simulink library "inp"  (Simulink 8.2)
settings: Solver=ode45  SolverMode=SingleTasking  StartTime=0.0  StopTime=10.0  RelTol=1e-3
undefined symbols (not defined in the file or in its folder): C_TICK_TIME_S, ENUM_FAULTSTATE_FAIL, ENUM_FAULTSTATE_INDETERMINATE, ENUM_FAULTSTATE_PASS, ENUM_KEYSWITCH_ON, FALSE, KINP_ABS1CANCommuOk_flg_ovrdflg, KINP_ABS1CANCommuOk_flg_ovrdval, KINP_ABS1CANPeriodTime_s, KINP_ABS1CANRolCntOk_flg_ovrdflg, KINP_ABS1CANRolCntOk_flg_ovrdval, KINP_ABS2CANChkSumOk_flg_ovrdflg, KINP_ABS2CANChkSumOk_flg_ovrdval, KINP_ABS2CANCommuOk_flg_ovrdflg, KINP_ABS2CANCommuOk_flg_ovrdval, KINP_ABS2CANPeriodTime_s, KINP_ABS2CANRolCntOk_flg_ovrdflg, KINP_ABS2CANRolCntOk_flg_ovrdval, KINP_ABS3CANCommuOk_flg_ovrdflg, KINP_ABS3CANCommuOk_flg_ovrdval, KINP_ABS4CANCommuOk_flg_ovrdflg, KINP_ABS4CANCommuOk_flg_ovrdval, KINP_ABSActive_flg_defval, KINP_ABSActive_flg_invalid, KINP_ABSActive_flg_ovrdflg, KINP_ABSActive_flg_ovrdval, KINP_ABSActive_flg_setvalid, KINP_ABSActive_flg_srvcflg, KINP_ABSActive_flg_srvcval, KINP_ABSCANAlive_flg_ovrdflg, KINP_ABSCANAlive_flg_ovrdval, KINP_ABSCANCommuOk_flg_ovrdflg, KINP_ABSCANCommuOk_flg_ovrdval, KINP_ABSCANValidOk_flg_ovrdflg, KINP_ABSCANValidOk_flg_ovrdval, KINP_ABSFault_flg_defval, KINP_ABSFault_flg_invalid, KINP_ABSFault_flg_ovrdflg, KINP_ABSFault_flg_ovrdval, KINP_ABSFault_flg_setvalid, KINP_ABSFault_flg_srvcflg, KINP_ABSFault_flg_srvcval, KINP_AC1CANCommuOk_flg_ovrdflg, KINP_AC1CANCommuOk_flg_ovrdval, KINP_ACCANAlive_flg_ovrdflg, KINP_ACCANAlive_flg_ovrdval, KINP_ACCANCommuOk_flg_ovrdflg, KINP_ACCANCommuOk_flg_ovrdval, KINP_ACCANValidOk_flg_ovrdflg, KINP_ACCANValidOk_flg_ovrdval, KINP_ACCCancelH_flg_defval, KINP_ACCCancelH_flg_invalid, KINP_ACCCancelH_flg_ovrdflg, KINP_ACCCancelH_flg_ovrdval, KINP_ACCCancelH_flg_setvalid, KINP_ACCCancelH_flg_srvcflg, KINP_ACCCancelH_flg_srvcval, KINP_AccelPedalPosn_pct_defval, KINP_AccelPedalPosn_pct_gain, KINP_AccelPedalPosn_pct_invalid ... (+1866)
libraries: inp x192, hcu_lib x34, simulink x33
(part 20 of 21)

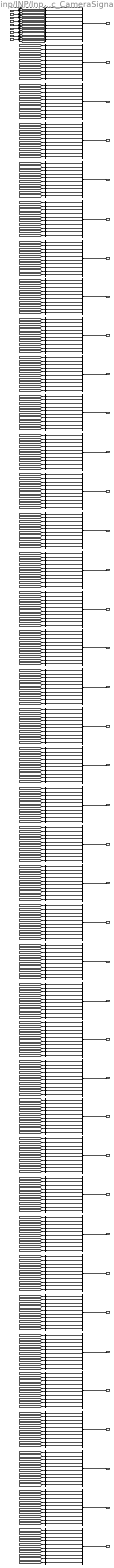
== system inp/INP/InputSignals/SignalProcess/Proc_CameraSignals ==
  VINP_Obstacle1_BUS_InHAL : Inport
  VINP_Obstacle2_BUS_InHAL : Inport  Port=2
  VINP_Obstacle3_BUS_InHAL : Inport  Port=3
  VINP_Obstacle4_BUS_InHAL : Inport  Port=4
  VINP_Obstacle5_BUS_InHAL : Inport  Port=5
  VINP_Obstacle6_BUS_InHAL : Inport  Port=6
  VINP_Obstacle7_BUS_InHAL : Inport  Port=7
  VINP_Obstacle8_BUS_InHAL : Inport  Port=8
  VINP_Obstacle9_BUS_InHAL : Inport  Port=9
  VINP_Obstacle10_BUS_InHAL : Inport  Port=10
  Bus Selector : BusSelector  OutputSignals=VINP_CamObs1ID_num_InHAL
  Bus Selector1 : BusSelector  OutputSignals=VINP_CamObs2ID_num_InHAL
  Bus Selector10 : BusSelector  OutputSignals=VINP_CamObs1IDValid_flg_InHAL
  Bus Selector100 : BusSelector  OutputSignals=VINP_CamObs1Status_enum_InHAL
  Bus Selector101 : BusSelector  OutputSignals=VINP_CamObs2Status_enum_InHAL
  Bus Selector102 : BusSelector  OutputSignals=VINP_CamObs3Status_enum_InHAL
  Bus Selector103 : BusSelector  OutputSignals=VINP_CamObs4Status_enum_InHAL
  Bus Selector104 : BusSelector  OutputSignals=VINP_CamObs5Status_enum_InHAL
  Bus Selector105 : BusSelector  OutputSignals=VINP_CamObs6Status_enum_InHAL
  Bus Selector106 : BusSelector  OutputSignals=VINP_CamObs7Status_enum_InHAL
  Bus Selector107 : BusSelector  OutputSignals=VINP_CamObs8Status_enum_InHAL
  Bus Selector108 : BusSelector  OutputSignals=VINP_CamObs9Status_enum_InHAL
  Bus Selector109 : BusSelector  OutputSignals=VINP_CamObs10Status_enum_InHAL
  Bus Selector11 : BusSelector  OutputSignals=VINP_CamObs2IDValid_flg_InHAL
  Bus Selector110 : BusSelector  OutputSignals=VINP_CamObs1StatusValid_flg_InHAL
  Bus Selector111 : BusSelector  OutputSignals=VINP_CamObs2StatusValid_flg_InHAL
  Bus Selector112 : BusSelector  OutputSignals=VINP_CamObs3StatusValid_flg_InHAL
  Bus Selector113 : BusSelector  OutputSignals=VINP_CamObs4StatusValid_flg_InHAL
  Bus Selector114 : BusSelector  OutputSignals=VINP_CamObs5StatusValid_flg_InHAL
  Bus Selector115 : BusSelector  OutputSignals=VINP_CamObs6StatusValid_flg_InHAL
  Bus Selector116 : BusSelector  OutputSignals=VINP_CamObs7StatusValid_flg_InHAL
  Bus Selector117 : BusSelector  OutputSignals=VINP_CamObs8StatusValid_flg_InHAL
  Bus Selector118 : BusSelector  OutputSignals=VINP_CamObs9StatusValid_flg_InHAL
  Bus Selector119 : BusSelector  OutputSignals=VINP_CamObs10StatusValid_flg_InHAL
  Bus Selector12 : BusSelector  OutputSignals=VINP_CamObs3IDValid_flg_InHAL
  Bus Selector120 : BusSelector  OutputSignals=VINP_CamObs1Valid_enum_InHAL
  Bus Selector121 : BusSelector  OutputSignals=VINP_CamObs2Valid_enum_InHAL
  Bus Selector122 : BusSelector  OutputSignals=VINP_CamObs3Valid_enum_InHAL
  Bus Selector123 : BusSelector  OutputSignals=VINP_CamObs4Valid_enum_InHAL
  Bus Selector124 : BusSelector  OutputSignals=VINP_CamObs5Valid_enum_InHAL
  Bus Selector125 : BusSelector  OutputSignals=VINP_CamObs6Valid_enum_InHAL
  Bus Selector126 : BusSelector  OutputSignals=VINP_CamObs7Valid_enum_InHAL
  Bus Selector127 : BusSelector  OutputSignals=VINP_CamObs8Valid_enum_InHAL
  Bus Selector128 : BusSelector  OutputSignals=VINP_CamObs9Valid_enum_InHAL
  Bus Selector129 : BusSelector  OutputSignals=VINP_CamObs10Valid_enum_InHAL
  Bus Selector13 : BusSelector  OutputSignals=VINP_CamObs4IDValid_flg_InHAL
  Bus Selector130 : BusSelector  OutputSignals=VINP_CamObs1ValidValid_flg_InHAL
  Bus Selector131 : BusSelector  OutputSignals=VINP_CamObs2ValidValid_flg_InHAL
  Bus Selector132 : BusSelector  OutputSignals=VINP_CamObs3ValidValid_flg_InHAL
  Bus Selector133 : BusSelector  OutputSignals=VINP_CamObs4ValidValid_flg_InHAL
  Bus Selector134 : BusSelector  OutputSignals=VINP_CamObs5ValidValid_flg_InHAL
  Bus Selector135 : BusSelector  OutputSignals=VINP_CamObs6ValidValid_flg_InHAL
  Bus Selector136 : BusSelector  OutputSignals=VINP_CamObs7ValidValid_flg_InHAL
  Bus Selector137 : BusSelector  OutputSignals=VINP_CamObs8ValidValid_flg_InHAL
  Bus Selector138 : BusSelector  OutputSignals=VINP_CamObs9ValidValid_flg_InHAL
  Bus Selector139 : BusSelector  OutputSignals=VINP_CamObs10ValidValid_flg_InHAL
  Bus Selector14 : BusSelector  OutputSignals=VINP_CamObs5IDValid_flg_InHAL
  Bus Selector140 : BusSelector  OutputSignals=VINP_CamObs1MoveInO_enum_InHAL
  Bus Selector141 : BusSelector  OutputSignals=VINP_CamObs2MoveInO_enum_InHAL
  Bus Selector142 : BusSelector  OutputSignals=VINP_CamObs3MoveInO_enum_InHAL
  Bus Selector143 : BusSelector  OutputSignals=VINP_CamObs4MoveInO_enum_InHAL
  Bus Selector144 : BusSelector  OutputSignals=VINP_CamObs5MoveInO_enum_InHAL
  Bus Selector145 : BusSelector  OutputSignals=VINP_CamObs6MoveInO_enum_InHAL
  Bus Selector146 : BusSelector  OutputSignals=VINP_CamObs7MoveInO_enum_InHAL
  Bus Selector147 : BusSelector  OutputSignals=VINP_CamObs8MoveInO_enum_InHAL
  Bus Selector148 : BusSelector  OutputSignals=VINP_CamObs9MoveInO_enum_InHAL
  Bus Selector149 : BusSelector  OutputSignals=VINP_CamObs10MoveInO_enum_InHAL
  Bus Selector15 : BusSelector  OutputSignals=VINP_CamObs6IDValid_flg_InHAL
  Bus Selector150 : BusSelector  OutputSignals=VINP_CamObs1MoveInOValid_flg_InHAL
  Bus Selector151 : BusSelector  OutputSignals=VINP_CamObs2MoveInOValid_flg_InHAL
  Bus Selector152 : BusSelector  OutputSignals=VINP_CamObs3MoveInOValid_flg_InHAL
  Bus Selector153 : BusSelector  OutputSignals=VINP_CamObs4MoveInOValid_flg_InHAL
  Bus Selector154 : BusSelector  OutputSignals=VINP_CamObs5MoveInOValid_flg_InHAL
  Bus Selector155 : BusSelector  OutputSignals=VINP_CamObs6MoveInOValid_flg_InHAL
  Bus Selector156 : BusSelector  OutputSignals=VINP_CamObs7MoveInOValid_flg_InHAL
  Bus Selector157 : BusSelector  OutputSignals=VINP_CamObs8MoveInOValid_flg_InHAL
  Bus Selector158 : BusSelector  OutputSignals=VINP_CamObs9MoveInOValid_flg_InHAL
  Bus Selector159 : BusSelector  OutputSignals=VINP_CamObs10MoveInOValid_flg_InHAL
  Bus Selector16 : BusSelector  OutputSignals=VINP_CamObs7IDValid_flg_InHAL
  Bus Selector160 : BusSelector  OutputSignals=VINP_CamObs1Blinker_enum_InHAL
  Bus Selector161 : BusSelector  OutputSignals=VINP_CamObs2Blinker_enum_InHAL
  Bus Selector162 : BusSelector  OutputSignals=VINP_CamObs3Blinker_enum_InHAL
  Bus Selector163 : BusSelector  OutputSignals=VINP_CamObs4Blinker_enum_InHAL
  Bus Selector164 : BusSelector  OutputSignals=VINP_CamObs5Blinker_enum_InHAL
  Bus Selector165 : BusSelector  OutputSignals=VINP_CamObs6Blinker_enum_InHAL
  Bus Selector166 : BusSelector  OutputSignals=VINP_CamObs7Blinker_enum_InHAL
  Bus Selector167 : BusSelector  OutputSignals=VINP_CamObs8Blinker_enum_InHAL
  Bus Selector168 : BusSelector  OutputSignals=VINP_CamObs9Blinker_enum_InHAL
  Bus Selector169 : BusSelector  OutputSignals=VINP_CamObs10Blinker_enum_InHAL
  Bus Selector17 : BusSelector  OutputSignals=VINP_CamObs8IDValid_flg_InHAL
  Bus Selector170 : BusSelector  OutputSignals=VINP_CamObs1BlinkerValid_flg_InHAL
  Bus Selector171 : BusSelector  OutputSignals=VINP_CamObs2BlinkerValid_flg_InHAL
  Bus Selector172 : BusSelector  OutputSignals=VINP_CamObs3BlinkerValid_flg_InHAL
  Bus Selector173 : BusSelector  OutputSignals=VINP_CamObs4BlinkerValid_flg_InHAL
  Bus Selector174 : BusSelector  OutputSignals=VINP_CamObs5BlinkerValid_flg_InHAL
  Bus Selector175 : BusSelector  OutputSignals=VINP_CamObs6BlinkerValid_flg_InHAL
  Bus Selector176 : BusSelector  OutputSignals=VINP_CamObs7BlinkerValid_flg_InHAL
  Bus Selector177 : BusSelector  OutputSignals=VINP_CamObs8BlinkerValid_flg_InHAL
  Bus Selector178 : BusSelector  OutputSignals=VINP_CamObs9BlinkerValid_flg_InHAL
  Bus Selector179 : BusSelector  OutputSignals=VINP_CamObs10BlinkerValid_flg_InHAL
  Bus Selector18 : BusSelector  OutputSignals=VINP_CamObs9IDValid_flg_InHAL
  Bus Selector180 : BusSelector  OutputSignals=VINP_CamObs1BrakeLight_flg_InHAL
  Bus Selector181 : BusSelector  OutputSignals=VINP_CamObs2BrakeLight_flg_InHAL
  Bus Selector182 : BusSelector  OutputSignals=VINP_CamObs3BrakeLight_flg_InHAL
  Bus Selector183 : BusSelector  OutputSignals=VINP_CamObs4BrakeLight_flg_InHAL
  Bus Selector184 : BusSelector  OutputSignals=VINP_CamObs5BrakeLight_flg_InHAL
  Bus Selector185 : BusSelector  OutputSignals=VINP_CamObs6BrakeLight_flg_InHAL
  Bus Selector186 : BusSelector  OutputSignals=VINP_CamObs7BrakeLight_flg_InHAL
  Bus Selector187 : BusSelector  OutputSignals=VINP_CamObs8BrakeLight_flg_InHAL
  Bus Selector188 : BusSelector  OutputSignals=VINP_CamObs9BrakeLight_flg_InHAL
  Bus Selector189 : BusSelector  OutputSignals=VINP_CamObs10BrakeLight_flg_InHAL
  Bus Selector19 : BusSelector  OutputSignals=VINP_CamObs10IDValid_flg_InHAL
  Bus Selector190 : BusSelector  OutputSignals=VINP_CamObs1BrakeLightValid_flg_InHAL
  Bus Selector191 : BusSelector  OutputSignals=VINP_CamObs2BrakeLightValid_flg_InHAL
  Bus Selector192 : BusSelector  OutputSignals=VINP_CamObs3BrakeLightValid_flg_InHAL
  Bus Selector193 : BusSelector  OutputSignals=VINP_CamObs4BrakeLightValid_flg_InHAL
  Bus Selector194 : BusSelector  OutputSignals=VINP_CamObs5BrakeLightValid_flg_InHAL
  Bus Selector195 : BusSelector  OutputSignals=VINP_CamObs6BrakeLightValid_flg_InHAL
  Bus Selector196 : BusSelector  OutputSignals=VINP_CamObs7BrakeLightValid_flg_InHAL
  Bus Selector197 : BusSelector  OutputSignals=VINP_CamObs8BrakeLightValid_flg_InHAL
  Bus Selector198 : BusSelector  OutputSignals=VINP_CamObs9BrakeLightValid_flg_InHAL
  Bus Selector199 : BusSelector  OutputSignals=VINP_CamObs10BrakeLightValid_flg_InHAL
  Bus Selector2 : BusSelector  OutputSignals=VINP_CamObs3ID_num_InHAL
  Bus Selector20 : BusSelector  OutputSignals=VINP_CamObs1PosX_m_InHAL
  Bus Selector200 : BusSelector  OutputSignals=VINP_CamObs1Length_m_InHAL
  Bus Selector201 : BusSelector  OutputSignals=VINP_CamObs1CIPVValid_flg_InHAL
  Bus Selector202 : BusSelector  OutputSignals=VINP_CamObs2CIPVValid_flg_InHAL
  Bus Selector203 : BusSelector  OutputSignals=VINP_CamObs3CIPVValid_flg_InHAL
  Bus Selector204 : BusSelector  OutputSignals=VINP_CamObs4CIPVValid_flg_InHAL
  Bus Selector205 : BusSelector  OutputSignals=VINP_CamObs5CIPVValid_flg_InHAL
  Bus Selector206 : BusSelector  OutputSignals=VINP_CamObs6CIPVValid_flg_InHAL
  Bus Selector207 : BusSelector  OutputSignals=VINP_CamObs7CIPVValid_flg_InHAL
  Bus Selector208 : BusSelector  OutputSignals=VINP_CamObs8CIPVValid_flg_InHAL
  Bus Selector209 : BusSelector  OutputSignals=VINP_CamObs9CIPVValid_flg_InHAL
  Bus Selector21 : BusSelector  OutputSignals=VINP_CamObs2PosX_m_InHAL
  Bus Selector210 : BusSelector  OutputSignals=VINP_CamObs10CIPVValid_flg_InHAL
  Bus Selector211 : BusSelector  OutputSignals=VINP_CamObs2Length_m_InHAL
  Bus Selector212 : BusSelector  OutputSignals=VINP_CamObs1VelY_mps_InHAL
  Bus Selector213 : BusSelector  OutputSignals=VINP_CamObs2VelY_mps_InHAL
  Bus Selector214 : BusSelector  OutputSignals=VINP_CamObs3VelY_mps_InHAL
  Bus Selector215 : BusSelector  OutputSignals=VINP_CamObs4VelY_mps_InHAL
  Bus Selector216 : BusSelector  OutputSignals=VINP_CamObs5VelY_mps_InHAL
  Bus Selector217 : BusSelector  OutputSignals=VINP_CamObs6VelY_mps_InHAL
  Bus Selector218 : BusSelector  OutputSignals=VINP_CamObs7VelY_mps_InHAL
  Bus Selector219 : BusSelector  OutputSignals=VINP_CamObs8VelY_mps_InHAL
  Bus Selector22 : BusSelector  OutputSignals=VINP_CamObs3PosX_m_InHAL
  Bus Selector220 : BusSelector  OutputSignals=VINP_CamObs9VelY_mps_InHAL
  Bus Selector221 : BusSelector  OutputSignals=VINP_CamObs10VelY_mps_InHAL
  Bus Selector222 : BusSelector  OutputSignals=VINP_CamObs3Length_m_InHAL
  Bus Selector223 : BusSelector  OutputSignals=VINP_CamObs1VelYValid_flg_InHAL
  Bus Selector224 : BusSelector  OutputSignals=VINP_CamObs2VelYValid_flg_InHAL
  Bus Selector225 : BusSelector  OutputSignals=VINP_CamObs3VelYValid_flg_InHAL
  Bus Selector226 : BusSelector  OutputSignals=VINP_CamObs4VelYValid_flg_InHAL
  Bus Selector227 : BusSelector  OutputSignals=VINP_CamObs5VelYValid_flg_InHAL
  Bus Selector228 : BusSelector  OutputSignals=VINP_CamObs6VelYValid_flg_InHAL
  Bus Selector229 : BusSelector  OutputSignals=VINP_CamObs7VelYValid_flg_InHAL
  Bus Selector23 : BusSelector  OutputSignals=VINP_CamObs4PosX_m_InHAL
  Bus Selector230 : BusSelector  OutputSignals=VINP_CamObs8VelYValid_flg_InHAL
  Bus Selector231 : BusSelector  OutputSignals=VINP_CamObs9VelYValid_flg_InHAL
  Bus Selector232 : BusSelector  OutputSignals=VINP_CamObs10VelYValid_flg_InHAL
  Bus Selector233 : BusSelector  OutputSignals=VINP_CamObs4Length_m_InHAL
  Bus Selector234 : BusSelector  OutputSignals=VINP_CamObs1AccX_mps2_InHAL
  Bus Selector235 : BusSelector  OutputSignals=VINP_CamObs2AccX_mps2_InHAL
  Bus Selector236 : BusSelector  OutputSignals=VINP_CamObs3AccX_mps2_InHAL
  Bus Selector237 : BusSelector  OutputSignals=VINP_CamObs4AccX_mps2_InHAL
  Bus Selector238 : BusSelector  OutputSignals=VINP_CamObs5AccX_mps2_InHAL
  Bus Selector239 : BusSelector  OutputSignals=VINP_CamObs6AccX_mps2_InHAL
  Bus Selector24 : BusSelector  OutputSignals=VINP_CamObs5PosX_m_InHAL
  Bus Selector240 : BusSelector  OutputSignals=VINP_CamObs7AccX_mps2_InHAL
  Bus Selector241 : BusSelector  OutputSignals=VINP_CamObs8AccX_mps2_InHAL
  Bus Selector242 : BusSelector  OutputSignals=VINP_CamObs9AccX_mps2_InHAL
  Bus Selector243 : BusSelector  OutputSignals=VINP_CamObs10AccX_mps2_InHAL
  Bus Selector244 : BusSelector  OutputSignals=VINP_CamObs5Length_m_InHAL
  Bus Selector245 : BusSelector  OutputSignals=VINP_CamObs1AccXValid_flg_InHAL
  Bus Selector246 : BusSelector  OutputSignals=VINP_CamObs2AccXValid_flg_InHAL
  Bus Selector247 : BusSelector  OutputSignals=VINP_CamObs3AccXValid_flg_InHAL
  Bus Selector248 : BusSelector  OutputSignals=VINP_CamObs4AccXValid_flg_InHAL
  Bus Selector249 : BusSelector  OutputSignals=VINP_CamObs5AccXValid_flg_InHAL
  Bus Selector25 : BusSelector  OutputSignals=VINP_CamObs6PosX_m_InHAL
  Bus Selector250 : BusSelector  OutputSignals=VINP_CamObs6AccXValid_flg_InHAL
  Bus Selector251 : BusSelector  OutputSignals=VINP_CamObs7AccXValid_flg_InHAL
  Bus Selector252 : BusSelector  OutputSignals=VINP_CamObs8AccXValid_flg_InHAL
  Bus Selector253 : BusSelector  OutputSignals=VINP_CamObs9AccXValid_flg_InHAL
  Bus Selector254 : BusSelector  OutputSignals=VINP_CamObs10AccXValid_flg_InHAL
  Bus Selector255 : BusSelector  OutputSignals=VINP_CamObs6Length_m_InHAL
  Bus Selector256 : BusSelector  OutputSignals=VINP_CamObs1Ang_deg_InHAL
  Bus Selector257 : BusSelector  OutputSignals=VINP_CamObs2Ang_deg_InHAL
  Bus Selector258 : BusSelector  OutputSignals=VINP_CamObs3Ang_deg_InHAL
  Bus Selector259 : BusSelector  OutputSignals=VINP_CamObs4Ang_deg_InHAL
  Bus Selector26 : BusSelector  OutputSignals=VINP_CamObs7PosX_m_InHAL
  Bus Selector260 : BusSelector  OutputSignals=VINP_CamObs5Ang_deg_InHAL
  Bus Selector261 : BusSelector  OutputSignals=VINP_CamObs6Ang_deg_InHAL
  Bus Selector262 : BusSelector  OutputSignals=VINP_CamObs7Ang_deg_InHAL
  Bus Selector263 : BusSelector  OutputSignals=VINP_CamObs8Ang_deg_InHAL
  Bus Selector264 : BusSelector  OutputSignals=VINP_CamObs9Ang_deg_InHAL
  Bus Selector265 : BusSelector  OutputSignals=VINP_CamObs10Ang_deg_InHAL
  Bus Selector266 : BusSelector  OutputSignals=VINP_CamObs7Length_m_InHAL
  Bus Selector267 : BusSelector  OutputSignals=VINP_CamObs1AngValid_flg_InHAL
  Bus Selector268 : BusSelector  OutputSignals=VINP_CamObs2AngValid_flg_InHAL
  Bus Selector269 : BusSelector  OutputSignals=VINP_CamObs3AngValid_flg_InHAL
  Bus Selector27 : BusSelector  OutputSignals=VINP_CamObs8PosX_m_InHAL
  Bus Selector270 : BusSelector  OutputSignals=VINP_CamObs4AngValid_flg_InHAL
  Bus Selector271 : BusSelector  OutputSignals=VINP_CamObs5AngValid_flg_InHAL
  Bus Selector272 : BusSelector  OutputSignals=VINP_CamObs6AngValid_flg_InHAL
  Bus Selector273 : BusSelector  OutputSignals=VINP_CamObs7AngValid_flg_InHAL
  Bus Selector274 : BusSelector  OutputSignals=VINP_CamObs8AngValid_flg_InHAL
  Bus Selector275 : BusSelector  OutputSignals=VINP_CamObs9AngValid_flg_InHAL
  Bus Selector276 : BusSelector  OutputSignals=VINP_CamObs10AngValid_flg_InHAL
  Bus Selector277 : BusSelector  OutputSignals=VINP_CamObs8Length_m_InHAL
  Bus Selector278 : BusSelector  OutputSignals=VINP_CamObs1AngRate_degps_InHAL
  Bus Selector279 : BusSelector  OutputSignals=VINP_CamObs2AngRate_degps_InHAL
  Bus Selector28 : BusSelector  OutputSignals=VINP_CamObs9PosX_m_InHAL
  Bus Selector280 : BusSelector  OutputSignals=VINP_CamObs3AngRate_degps_InHAL
  Bus Selector281 : BusSelector  OutputSignals=VINP_CamObs4AngRate_degps_InHAL
  Bus Selector282 : BusSelector  OutputSignals=VINP_CamObs5AngRate_degps_InHAL
  Bus Selector283 : BusSelector  OutputSignals=VINP_CamObs6AngRate_degps_InHAL
  Bus Selector284 : BusSelector  OutputSignals=VINP_CamObs7AngRate_degps_InHAL
  Bus Selector285 : BusSelector  OutputSignals=VINP_CamObs8AngRate_degps_InHAL
  Bus Selector286 : BusSelector  OutputSignals=VINP_CamObs9AngRate_degps_InHAL
  Bus Selector287 : BusSelector  OutputSignals=VINP_CamObs10AngRate_degps_InHAL
  Bus Selector288 : BusSelector  OutputSignals=VINP_CamObs9Length_m_InHAL
  Bus Selector289 : BusSelector  OutputSignals=VINP_CamObs1AngRateValid_flg_InHAL
  Bus Selector29 : BusSelector  OutputSignals=VINP_CamObs10PosX_m_InHAL
  Bus Selector290 : BusSelector  OutputSignals=VINP_CamObs2AngRateValid_flg_InHAL
  Bus Selector291 : BusSelector  OutputSignals=VINP_CamObs3AngRateValid_flg_InHAL
  Bus Selector292 : BusSelector  OutputSignals=VINP_CamObs4AngRateValid_flg_InHAL
  Bus Selector293 : BusSelector  OutputSignals=VINP_CamObs5AngRateValid_flg_InHAL
  Bus Selector294 : BusSelector  OutputSignals=VINP_CamObs6AngRateValid_flg_InHAL
  Bus Selector295 : BusSelector  OutputSignals=VINP_CamObs7AngRateValid_flg_InHAL
  Bus Selector296 : BusSelector  OutputSignals=VINP_CamObs8AngRateValid_flg_InHAL
  Bus Selector297 : BusSelector  OutputSignals=VINP_CamObs9AngRateValid_flg_InHAL
  Bus Selector298 : BusSelector  OutputSignals=VINP_CamObs10AngRateValid_flg_InHAL
  Bus Selector299 : BusSelector  OutputSignals=VINP_CamObs10Length_m_InHAL
  Bus Selector3 : BusSelector  OutputSignals=VINP_CamObs4ID_num_InHAL
  Bus Selector30 : BusSelector  OutputSignals=VINP_CamObs1PosXValid_flg_InHAL
  Bus Selector300 : BusSelector  OutputSignals=VINP_CamObs1Replaced_flg_InHAL
  Bus Selector301 : BusSelector  OutputSignals=VINP_CamObs2Replaced_flg_InHAL
  Bus Selector302 : BusSelector  OutputSignals=VINP_CamObs3Replaced_flg_InHAL
  Bus Selector303 : BusSelector  OutputSignals=VINP_CamObs4Replaced_flg_InHAL
  Bus Selector304 : BusSelector  OutputSignals=VINP_CamObs5Replaced_flg_InHAL
  Bus Selector305 : BusSelector  OutputSignals=VINP_CamObs6Replaced_flg_InHAL
  Bus Selector306 : BusSelector  OutputSignals=VINP_CamObs7Replaced_flg_InHAL
  Bus Selector307 : BusSelector  OutputSignals=VINP_CamObs8Replaced_flg_InHAL
  Bus Selector308 : BusSelector  OutputSignals=VINP_CamObs9Replaced_flg_InHAL
  Bus Selector309 : BusSelector  OutputSignals=VINP_CamObs10Replaced_flg_InHAL
  Bus Selector31 : BusSelector  OutputSignals=VINP_CamObs2PosXValid_flg_InHAL
  Bus Selector310 : BusSelector  OutputSignals=VINP_CamObs1LengthValid_flg_InHAL
  Bus Selector311 : BusSelector  OutputSignals=VINP_CamObs2LengthValid_flg_InHAL
  Bus Selector312 : BusSelector  OutputSignals=VINP_CamObs3LengthValid_flg_InHAL
  Bus Selector313 : BusSelector  OutputSignals=VINP_CamObs4LengthValid_flg_InHAL
  Bus Selector314 : BusSelector  OutputSignals=VINP_CamObs5LengthValid_flg_InHAL
  Bus Selector315 : BusSelector  OutputSignals=VINP_CamObs6LengthValid_flg_InHAL
  Bus Selector316 : BusSelector  OutputSignals=VINP_CamObs7LengthValid_flg_InHAL
  Bus Selector317 : BusSelector  OutputSignals=VINP_CamObs8LengthValid_flg_InHAL
  Bus Selector318 : BusSelector  OutputSignals=VINP_CamObs9LengthValid_flg_InHAL
  Bus Selector319 : BusSelector  OutputSignals=VINP_CamObs10LengthValid_flg_InHAL
  Bus Selector32 : BusSelector  OutputSignals=VINP_CamObs3PosXValid_flg_InHAL
  Bus Selector320 : BusSelector  OutputSignals=VINP_CamObs1Width_m_InHAL
  Bus Selector321 : BusSelector  OutputSignals=VINP_CamObs2Width_m_InHAL
  Bus Selector322 : BusSelector  OutputSignals=VINP_CamObs3Width_m_InHAL
  Bus Selector323 : BusSelector  OutputSignals=VINP_CamObs4Width_m_InHAL
  Bus Selector324 : BusSelector  OutputSignals=VINP_CamObs5Width_m_InHAL
  Bus Selector325 : BusSelector  OutputSignals=VINP_CamObs6Width_m_InHAL
  Bus Selector326 : BusSelector  OutputSignals=VINP_CamObs7Width_m_InHAL
  Bus Selector327 : BusSelector  OutputSignals=VINP_CamObs8Width_m_InHAL
  Bus Selector328 : BusSelector  OutputSignals=VINP_CamObs9Width_m_InHAL
  Bus Selector329 : BusSelector  OutputSignals=VINP_CamObs10Width_m_InHAL
  Bus Selector33 : BusSelector  OutputSignals=VINP_CamObs4PosXValid_flg_InHAL
  Bus Selector330 : BusSelector  OutputSignals=VINP_CamObs1WidthValid_flg_InHAL
  Bus Selector331 : BusSelector  OutputSignals=VINP_CamObs2WidthValid_flg_InHAL
  Bus Selector332 : BusSelector  OutputSignals=VINP_CamObs3WidthValid_flg_InHAL
  Bus Selector333 : BusSelector  OutputSignals=VINP_CamObs4WidthValid_flg_InHAL
  Bus Selector334 : BusSelector  OutputSignals=VINP_CamObs5WidthValid_flg_InHAL
  Bus Selector335 : BusSelector  OutputSignals=VINP_CamObs6WidthValid_flg_InHAL
  Bus Selector336 : BusSelector  OutputSignals=VINP_CamObs7WidthValid_flg_InHAL
  Bus Selector337 : BusSelector  OutputSignals=VINP_CamObs8WidthValid_flg_InHAL
  Bus Selector338 : BusSelector  OutputSignals=VINP_CamObs9WidthValid_flg_InHAL
  Bus Selector339 : BusSelector  OutputSignals=VINP_CamObs10WidthValid_flg_InHAL
  Bus Selector34 : BusSelector  OutputSignals=VINP_CamObs5PosXValid_flg_InHAL
  Bus Selector340 : BusSelector  OutputSignals=VINP_CamObs1Age_cnt_InHAL
  Bus Selector341 : BusSelector  OutputSignals=VINP_CamObs2Age_cnt_InHAL
  Bus Selector342 : BusSelector  OutputSignals=VINP_CamObs3Age_cnt_InHAL
  Bus Selector343 : BusSelector  OutputSignals=VINP_CamObs4Age_cnt_InHAL
  Bus Selector344 : BusSelector  OutputSignals=VINP_CamObs5Age_cnt_InHAL
  Bus Selector345 : BusSelector  OutputSignals=VINP_CamObs6Age_cnt_InHAL
  Bus Selector346 : BusSelector  OutputSignals=VINP_CamObs7Age_cnt_InHAL
  Bus Selector347 : BusSelector  OutputSignals=VINP_CamObs8Age_cnt_InHAL
  Bus Selector348 : BusSelector  OutputSignals=VINP_CamObs9Age_cnt_InHAL
  Bus Selector349 : BusSelector  OutputSignals=VINP_CamObs10Age_cnt_InHAL
  Bus Selector35 : BusSelector  OutputSignals=VINP_CamObs6PosXValid_flg_InHAL
  Bus Selector350 : BusSelector  OutputSignals=VINP_CamObs1AgeValid_flg_InHAL
  Bus Selector351 : BusSelector  OutputSignals=VINP_CamObs2AgeValid_flg_InHAL
  Bus Selector352 : BusSelector  OutputSignals=VINP_CamObs3AgeValid_flg_InHAL
  Bus Selector353 : BusSelector  OutputSignals=VINP_CamObs4AgeValid_flg_InHAL
  Bus Selector354 : BusSelector  OutputSignals=VINP_CamObs5AgeValid_flg_InHAL
  Bus Selector355 : BusSelector  OutputSignals=VINP_CamObs6AgeValid_flg_InHAL
  Bus Selector356 : BusSelector  OutputSignals=VINP_CamObs7AgeValid_flg_InHAL
  Bus Selector357 : BusSelector  OutputSignals=VINP_CamObs8AgeValid_flg_InHAL
  Bus Selector358 : BusSelector  OutputSignals=VINP_CamObs9AgeValid_flg_InHAL
  Bus Selector359 : BusSelector  OutputSignals=VINP_CamObs10AgeValid_flg_InHAL
  Bus Selector36 : BusSelector  OutputSignals=VINP_CamObs7PosXValid_flg_InHAL
  Bus Selector360 : BusSelector  OutputSignals=VINP_CamObs1Lane_enum_InHAL
  Bus Selector361 : BusSelector  OutputSignals=VINP_CamObs2Lane_enum_InHAL
  Bus Selector362 : BusSelector  OutputSignals=VINP_CamObs3Lane_enum_InHAL
  Bus Selector363 : BusSelector  OutputSignals=VINP_CamObs4Lane_enum_InHAL
  Bus Selector364 : BusSelector  OutputSignals=VINP_CamObs5Lane_enum_InHAL
  Bus Selector365 : BusSelector  OutputSignals=VINP_CamObs6Lane_enum_InHAL
  Bus Selector366 : BusSelector  OutputSignals=VINP_CamObs7Lane_enum_InHAL
  Bus Selector367 : BusSelector  OutputSignals=VINP_CamObs8Lane_enum_InHAL
  Bus Selector368 : BusSelector  OutputSignals=VINP_CamObs9Lane_enum_InHAL
  Bus Selector369 : BusSelector  OutputSignals=VINP_CamObs10Lane_enum_InHAL
  Bus Selector37 : BusSelector  OutputSignals=VINP_CamObs8PosXValid_flg_InHAL
  Bus Selector370 : BusSelector  OutputSignals=VINP_CamObs1LaneValid_flg_InHAL
  Bus Selector371 : BusSelector  OutputSignals=VINP_CamObs2LaneValid_flg_InHAL
  Bus Selector372 : BusSelector  OutputSignals=VINP_CamObs3LaneValid_flg_InHAL
  Bus Selector373 : BusSelector  OutputSignals=VINP_CamObs4LaneValid_flg_InHAL
  Bus Selector374 : BusSelector  OutputSignals=VINP_CamObs5LaneValid_flg_InHAL
  Bus Selector375 : BusSelector  OutputSignals=VINP_CamObs6LaneValid_flg_InHAL
  Bus Selector376 : BusSelector  OutputSignals=VINP_CamObs7LaneValid_flg_InHAL
  Bus Selector377 : BusSelector  OutputSignals=VINP_CamObs8LaneValid_flg_InHAL
  Bus Selector378 : BusSelector  OutputSignals=VINP_CamObs9LaneValid_flg_InHAL
  Bus Selector379 : BusSelector  OutputSignals=VINP_CamObs10LaneValid_flg_InHAL
  Bus Selector38 : BusSelector  OutputSignals=VINP_CamObs9PosXValid_flg_InHAL
  Bus Selector380 : BusSelector  OutputSignals=VINP_CamObs1CIPV_flg_InHAL
  Bus Selector381 : BusSelector  OutputSignals=VINP_CamObs2CIPV_flg_InHAL
  Bus Selector382 : BusSelector  OutputSignals=VINP_CamObs3CIPV_flg_InHAL
  Bus Selector383 : BusSelector  OutputSignals=VINP_CamObs4CIPV_flg_InHAL
  Bus Selector384 : BusSelector  OutputSignals=VINP_CamObs5CIPV_flg_InHAL
  Bus Selector385 : BusSelector  OutputSignals=VINP_CamObs6CIPV_flg_InHAL
  Bus Selector386 : BusSelector  OutputSignals=VINP_CamObs7CIPV_flg_InHAL
  Bus Selector387 : BusSelector  OutputSignals=VINP_CamObs8CIPV_flg_InHAL
  Bus Selector388 : BusSelector  OutputSignals=VINP_CamObs9CIPV_flg_InHAL
  Bus Selector389 : BusSelector  OutputSignals=VINP_CamObs10CIPV_flg_InHAL
  Bus Selector39 : BusSelector  OutputSignals=VINP_CamObs10PosXValid_flg_InHAL
  Bus Selector390 : BusSelector  OutputSignals=VINP_CamObs1ReplacedValid_flg_InHAL
  Bus Selector391 : BusSelector  OutputSignals=VINP_CamObs2ReplacedValid_flg_InHAL
  Bus Selector392 : BusSelector  OutputSignals=VINP_CamObs3ReplacedValid_flg_InHAL
  Bus Selector393 : BusSelector  OutputSignals=VINP_CamObs4ReplacedValid_flg_InHAL
  Bus Selector394 : BusSelector  OutputSignals=VINP_CamObs5ReplacedValid_flg_InHAL
  Bus Selector395 : BusSelector  OutputSignals=VINP_CamObs6ReplacedValid_flg_InHAL
  Bus Selector396 : BusSelector  OutputSignals=VINP_CamObs7ReplacedValid_flg_InHAL
  Bus Selector397 : BusSelector  OutputSignals=VINP_CamObs8ReplacedValid_flg_InHAL
  Bus Selector398 : BusSelector  OutputSignals=VINP_CamObs9ReplacedValid_flg_InHAL
  Bus Selector399 : BusSelector  OutputSignals=VINP_CamObs10ReplacedValid_flg_InHAL
  Bus Selector4 : BusSelector  OutputSignals=VINP_CamObs5ID_num_InHAL
  Bus Selector40 : BusSelector  OutputSignals=VINP_CamObs1PosY_m_InHAL
  Bus Selector41 : BusSelector  OutputSignals=VINP_CamObs2PosY_m_InHAL
  Bus Selector42 : BusSelector  OutputSignals=VINP_CamObs3PosY_m_InHAL
  Bus Selector43 : BusSelector  OutputSignals=VINP_CamObs4PosY_m_InHAL
  Bus Selector44 : BusSelector  OutputSignals=VINP_CamObs5PosY_m_InHAL
  Bus Selector45 : BusSelector  OutputSignals=VINP_CamObs6PosY_m_InHAL
  Bus Selector46 : BusSelector  OutputSignals=VINP_CamObs7PosY_m_InHAL
  Bus Selector47 : BusSelector  OutputSignals=VINP_CamObs8PosY_m_InHAL
  Bus Selector48 : BusSelector  OutputSignals=VINP_CamObs9PosY_m_InHAL
  Bus Selector49 : BusSelector  OutputSignals=VINP_CamObs10PosY_m_InHAL
  Bus Selector5 : BusSelector  OutputSignals=VINP_CamObs6ID_num_InHAL
  Bus Selector50 : BusSelector  OutputSignals=VINP_CamObs1PosYValid_flg_InHAL
  Bus Selector51 : BusSelector  OutputSignals=VINP_CamObs2PosYValid_flg_InHAL
  Bus Selector52 : BusSelector  OutputSignals=VINP_CamObs3PosYValid_flg_InHAL
  Bus Selector53 : BusSelector  OutputSignals=VINP_CamObs4PosYValid_flg_InHAL
  Bus Selector54 : BusSelector  OutputSignals=VINP_CamObs5PosYValid_flg_InHAL
  Bus Selector55 : BusSelector  OutputSignals=VINP_CamObs6PosYValid_flg_InHAL
  Bus Selector56 : BusSelector  OutputSignals=VINP_CamObs7PosYValid_flg_InHAL
  Bus Selector57 : BusSelector  OutputSignals=VINP_CamObs8PosYValid_flg_InHAL
  Bus Selector58 : BusSelector  OutputSignals=VINP_CamObs9PosYValid_flg_InHAL
  Bus Selector59 : BusSelector  OutputSignals=VINP_CamObs10PosYValid_flg_InHAL
  Bus Selector6 : BusSelector  OutputSignals=VINP_CamObs7ID_num_InHAL
  Bus Selector60 : BusSelector  OutputSignals=VINP_CamObs1VelX_mps_InHAL
  Bus Selector61 : BusSelector  OutputSignals=VINP_CamObs2VelX_mps_InHAL
  Bus Selector62 : BusSelector  OutputSignals=VINP_CamObs3VelX_mps_InHAL
  Bus Selector63 : BusSelector  OutputSignals=VINP_CamObs4VelX_mps_InHAL
  Bus Selector64 : BusSelector  OutputSignals=VINP_CamObs5VelX_mps_InHAL
  Bus Selector65 : BusSelector  OutputSignals=VINP_CamObs6VelX_mps_InHAL
  Bus Selector66 : BusSelector  OutputSignals=VINP_CamObs7VelX_mps_InHAL
  Bus Selector67 : BusSelector  OutputSignals=VINP_CamObs8VelX_mps_InHAL
  Bus Selector68 : BusSelector  OutputSignals=VINP_CamObs9VelX_mps_InHAL
  Bus Selector69 : BusSelector  OutputSignals=VINP_CamObs10VelX_mps_InHAL
  Bus Selector7 : BusSelector  OutputSignals=VINP_CamObs8ID_num_InHAL
  Bus Selector70 : BusSelector  OutputSignals=VINP_CamObs1VelXValid_flg_InHAL
  Bus Selector71 : BusSelector  OutputSignals=VINP_CamObs2VelXValid_flg_InHAL
  Bus Selector72 : BusSelector  OutputSignals=VINP_CamObs3VelXValid_flg_InHAL
  Bus Selector73 : BusSelector  OutputSignals=VINP_CamObs4VelXValid_flg_InHAL
  Bus Selector74 : BusSelector  OutputSignals=VINP_CamObs5VelXValid_flg_InHAL
  Bus Selector75 : BusSelector  OutputSignals=VINP_CamObs6VelXValid_flg_InHAL
  Bus Selector76 : BusSelector  OutputSignals=VINP_CamObs7VelXValid_flg_InHAL
  Bus Selector77 : BusSelector  OutputSignals=VINP_CamObs8VelXValid_flg_InHAL
  Bus Selector78 : BusSelector  OutputSignals=VINP_CamObs9VelXValid_flg_InHAL
  Bus Selector79 : BusSelector  OutputSignals=VINP_CamObs10VelXValid_flg_InHAL
  Bus Selector8 : BusSelector  OutputSignals=VINP_CamObs9ID_num_InHAL
  Bus Selector80 : BusSelector  OutputSignals=VINP_CamObs1Type_enum_InHAL
  Bus Selector81 : BusSelector  OutputSignals=VINP_CamObs2Type_enum_InHAL
  Bus Selector82 : BusSelector  OutputSignals=VINP_CamObs3Type_enum_InHAL
  Bus Selector83 : BusSelector  OutputSignals=VINP_CamObs4Type_enum_InHAL
  Bus Selector84 : BusSelector  OutputSignals=VINP_CamObs5Type_enum_InHAL
  Bus Selector85 : BusSelector  OutputSignals=VINP_CamObs6Type_enum_InHAL
  Bus Selector86 : BusSelector  OutputSignals=VINP_CamObs7Type_enum_InHAL
  Bus Selector87 : BusSelector  OutputSignals=VINP_CamObs8Type_enum_InHAL
  Bus Selector88 : BusSelector  OutputSignals=VINP_CamObs9Type_enum_InHAL
  Bus Selector89 : BusSelector  OutputSignals=VINP_CamObs10Type_enum_InHAL
  Bus Selector9 : BusSelector  OutputSignals=VINP_CamObs10ID_num_InHAL
  Bus Selector90 : BusSelector  OutputSignals=VINP_CamObs1TypeValid_flg_InHAL
  Bus Selector91 : BusSelector  OutputSignals=VINP_CamObs2TypeValid_flg_InHAL
  Bus Selector92 : BusSelector  OutputSignals=VINP_CamObs3TypeValid_flg_InHAL
  Bus Selector93 : BusSelector  OutputSignals=VINP_CamObs4TypeValid_flg_InHAL
  Bus Selector94 : BusSelector  OutputSignals=VINP_CamObs5TypeValid_flg_InHAL
  Bus Selector95 : BusSelector  OutputSignals=VINP_CamObs6TypeValid_flg_InHAL
  Bus Selector96 : BusSelector  OutputSignals=VINP_CamObs7TypeValid_flg_InHAL
  Bus Selector97 : BusSelector  OutputSignals=VINP_CamObs8TypeValid_flg_InHAL
  Bus Selector98 : BusSelector  OutputSignals=VINP_CamObs9TypeValid_flg_InHAL
  Bus Selector99 : BusSelector  OutputSignals=VINP_CamObs10TypeValid_flg_InHAL
  Mux{,1..39} (40 alike) : Mux  Inputs=10
  VINP_CamObsID10_num_InProc : Outport
  VINP_CamObsIDValid10_flg_InProc : Outport  Port=2
  VINP_CamObsPosX10_m_InProc : Outport  Port=3
  VINP_CamObsPosXValid10_flg_InProc : Outport  Port=4
  VINP_CamObsPosY10_m_InProc : Outport  Port=5
  VINP_CamObsPosYValid10_flg_InProc : Outport  Port=6
  VINP_CamObsVelX10_mps_InProc : Outport  Port=7
  VINP_CamObsVelXValid10_flg_InProc : Outport  Port=8
  VINP_CamObsType10_enum_InProc : Outport  Port=9
  VINP_CamObsTypeValid10_flg_InProc : Outport  Port=10
  VINP_CamObsStatus10_enum_InProc : Outport  Port=11
  VINP_CamObsStatusValid10_flg_InProc : Outport  Port=12
  VINP_CamObsValid10_enum_InProc : Outport  Port=13
  VINP_CamObsValidValid10_flg_InProc : Outport  Port=14
  VINP_CamObsMoveInO10_enum_InProc : Outport  Port=15
  VINP_CamObsMoveInOValid10_flg_InProc : Outport  Port=16
  VINP_CamObsBlinker10_enum_InProc : Outport  Port=17
  VINP_CamObsBlinkerValid10_flg_InProc : Outport  Port=18
  VINP_CamObsBrakeLight10_flg_InProc : Outport  Port=19
  VINP_CamObsBrakeLightValid10_flg_InProc : Outport  Port=20
  VINP_CamObsLength10_m_InProc : Outport  Port=21
  VINP_CamObsLengthValid10_flg_InProc : Outport  Port=22
  VINP_CamObsWidth10_m_InProc : Outport  Port=23
  VINP_CamObsWidthValid10_flg_InProc : Outport  Port=24
  VINP_CamObsAge10_cnt_InProc : Outport  Port=25
  VINP_CamObsAgeValid10_flg_InProc : Outport  Port=26
  VINP_CamObsLane10_enum_InProc : Outport  Port=27
  VINP_CamObsLaneValid10_flg_InProc : Outport  Port=28
  VINP_CamObsCIPV10_flg_InProc : Outport  Port=29
  VINP_CamObsCIPVValid10_flg_InProc : Outport  Port=30
  VINP_CamObsVelY10_mps_InProc : Outport  Port=31
  VINP_CamObsVelYValid10_flg_InProc : Outport  Port=32
  VINP_CamObsAccX10_mps2_InProc : Outport  Port=33
  VINP_CamObsAccXValid10_flg_InProc : Outport  Port=34
  VINP_CamObsAng10_deg_InProc : Outport  Port=35
  VINP_CamObsAngValid10_flg_InProc : Outport  Port=36
  VINP_CamObsAngRate10_degps_InProc : Outport  Port=37
  VINP_CamObsAngRateValid10_flg_InProc : Outport  Port=38
  VINP_CamObsReplaced10_flg_InProc : Outport  Port=39
  VINP_CamObsReplacedValid10_flg_InProc : Outport  Port=40
  wires:
  VINP_Obstacle1_BUS_InHAL/1 -> [VINP_Obstacle1_BUS_InHAL], Bus Selector/1
  VINP_Obstacle2_BUS_InHAL/1 -> [VINP_Obstacle2_BUS_InHAL], Bus Selector1/1
  VINP_Obstacle3_BUS_InHAL/1 -> [VINP_Obstacle3_BUS_InHAL], Bus Selector2/1
  VINP_Obstacle4_BUS_InHAL/1 -> [VINP_Obstacle4_BUS_InHAL], Bus Selector3/1
  VINP_Obstacle5_BUS_InHAL/1 -> [VINP_Obstacle5_BUS_InHAL], Bus Selector4/1
  VINP_Obstacle6_BUS_InHAL/1 -> Bus Selector5/1, [VINP_Obstacle6_BUS_InHAL]
  VINP_Obstacle7_BUS_InHAL/1 -> [VINP_Obstacle7_BUS_InHAL], Bus Selector6/1
  VINP_Obstacle8_BUS_InHAL/1 -> Bus Selector7/1, [VINP_Obstacle8_BUS_InHAL]
  VINP_Obstacle9_BUS_InHAL/1 -> [VINP_Obstacle9_BUS_InHAL], Bus Selector8/1
  VINP_Obstacle10_BUS_InHAL/1 -> Bus Selector9/1, [VINP_Obstacle10_BUS_InHAL]
  Bus Selector/1 -> Mux/1  "<VINP_CamObs1ID_num_InHAL>"
  Bus Selector1/1 -> Mux/2  "<VINP_CamObs2ID_num_InHAL>"
  Bus Selector2/1 -> Mux/3  "<VINP_CamObs3ID_num_InHAL>"
  Bus Selector3/1 -> Mux/4  "<VINP_CamObs4ID_num_InHAL>"
  Bus Selector4/1 -> Mux/5  "<VINP_CamObs5ID_num_InHAL>"
  Bus Selector5/1 -> Mux/6  "<VINP_CamObs6ID_num_InHAL>"
  Bus Selector6/1 -> Mux/7  "<VINP_CamObs7ID_num_InHAL>"
  Bus Selector7/1 -> Mux/8  "<VINP_CamObs8ID_num_InHAL>"
  Bus Selector8/1 -> Mux/9  "<VINP_CamObs9ID_num_InHAL>"
  Bus Selector9/1 -> Mux/10  "<VINP_CamObs10ID_num_InHAL>"
  Mux/1 -> VINP_CamObsID10_num_InProc/1  "VINP_CamObsID10_num_InProc"
  Bus Selector10/1 -> Mux1/1  "<VINP_CamObs1IDValid_flg_InHAL>"
  Bus Selector11/1 -> Mux1/2  "<VINP_CamObs2IDValid_flg_InHAL>"
  Bus Selector12/1 -> Mux1/3  "<VINP_CamObs3IDValid_flg_InHAL>"
  Bus Selector13/1 -> Mux1/4  "<VINP_CamObs4IDValid_flg_InHAL>"
  Bus Selector14/1 -> Mux1/5  "<VINP_CamObs5IDValid_flg_InHAL>"
  Bus Selector15/1 -> Mux1/6  "<VINP_CamObs6IDValid_flg_InHAL>"
  Bus Selector16/1 -> Mux1/7  "<VINP_CamObs7IDValid_flg_InHAL>"
  Bus Selector17/1 -> Mux1/8  "<VINP_CamObs8IDValid_flg_InHAL>"
  Bus Selector18/1 -> Mux1/9  "<VINP_CamObs9IDValid_flg_InHAL>"
  [VINP_Obstacle1_BUS_InHAL] -> Bus Selector10/1
  [VINP_Obstacle2_BUS_InHAL] -> Bus Selector11/1
  [VINP_Obstacle3_BUS_InHAL] -> Bus Selector12/1
  [VINP_Obstacle4_BUS_InHAL] -> Bus Selector13/1
  [VINP_Obstacle5_BUS_InHAL] -> Bus Selector14/1
  [VINP_Obstacle6_BUS_InHAL] -> Bus Selector15/1
  [VINP_Obstacle7_BUS_InHAL] -> Bus Selector16/1
  [VINP_Obstacle8_BUS_InHAL] -> Bus Selector17/1
  [VINP_Obstacle9_BUS_InHAL] -> Bus Selector18/1
  [VINP_Obstacle10_BUS_InHAL] -> Bus Selector19/1
  Bus Selector19/1 -> Mux1/10  "<VINP_CamObs10IDValid_flg_InHAL>"
  Mux1/1 -> VINP_CamObsIDValid10_flg_InProc/1  "VINP_CamObsIDValid10_flg_InProc"
  Bus Selector20/1 -> Mux2/1  "<VINP_CamObs1PosX_m_InHAL>"
  Bus Selector21/1 -> Mux2/2  "<VINP_CamObs2PosX_m_InHAL>"
  Bus Selector22/1 -> Mux2/3  "<VINP_CamObs3PosX_m_InHAL>"
  Bus Selector23/1 -> Mux2/4  "<VINP_CamObs4PosX_m_InHAL>"
  Bus Selector24/1 -> Mux2/5  "<VINP_CamObs5PosX_m_InHAL>"
  Bus Selector25/1 -> Mux2/6  "<VINP_CamObs6PosX_m_InHAL>"
  Bus Selector26/1 -> Mux2/7  "<VINP_CamObs7PosX_m_InHAL>"
  Bus Selector27/1 -> Mux2/8  "<VINP_CamObs8PosX_m_InHAL>"
  Bus Selector28/1 -> Mux2/9  "<VINP_CamObs9PosX_m_InHAL>"
  [VINP_Obstacle1_BUS_InHAL] -> Bus Selector20/1
  [VINP_Obstacle2_BUS_InHAL] -> Bus Selector21/1
  [VINP_Obstacle3_BUS_InHAL] -> Bus Selector22/1
  [VINP_Obstacle4_BUS_InHAL] -> Bus Selector23/1
  [VINP_Obstacle5_BUS_InHAL] -> Bus Selector24/1
  [VINP_Obstacle6_BUS_InHAL] -> Bus Selector25/1
  [VINP_Obstacle7_BUS_InHAL] -> Bus Selector26/1
  [VINP_Obstacle8_BUS_InHAL] -> Bus Selector27/1
  [VINP_Obstacle9_BUS_InHAL] -> Bus Selector28/1
  [VINP_Obstacle10_BUS_InHAL] -> Bus Selector29/1
  Bus Selector29/1 -> Mux2/10  "<VINP_CamObs10PosX_m_InHAL>"
  Mux2/1 -> VINP_CamObsPosX10_m_InProc/1  "VINP_CamObsPosX10_m_InProc"
  Bus Selector30/1 -> Mux3/1  "<VINP_CamObs1PosXValid_flg_InHAL>"
  Bus Selector31/1 -> Mux3/2  "<VINP_CamObs2PosXValid_flg_InHAL>"
  Bus Selector32/1 -> Mux3/3  "<VINP_CamObs3PosXValid_flg_InHAL>"
  Bus Selector33/1 -> Mux3/4  "<VINP_CamObs4PosXValid_flg_InHAL>"
  Bus Selector34/1 -> Mux3/5  "<VINP_CamObs5PosXValid_flg_InHAL>"
  Bus Selector35/1 -> Mux3/6  "<VINP_CamObs6PosXValid_flg_InHAL>"
  Bus Selector36/1 -> Mux3/7  "<VINP_CamObs7PosXValid_flg_InHAL>"
  Bus Selector37/1 -> Mux3/8  "<VINP_CamObs8PosXValid_flg_InHAL>"
  Bus Selector38/1 -> Mux3/9  "<VINP_CamObs9PosXValid_flg_InHAL>"
  [VINP_Obstacle1_BUS_InHAL] -> Bus Selector30/1
  [VINP_Obstacle2_BUS_InHAL] -> Bus Selector31/1
  [VINP_Obstacle3_BUS_InHAL] -> Bus Selector32/1
  [VINP_Obstacle4_BUS_InHAL] -> Bus Selector33/1
  [VINP_Obstacle5_BUS_InHAL] -> Bus Selector34/1
  [VINP_Obstacle6_BUS_InHAL] -> Bus Selector35/1
  [VINP_Obstacle7_BUS_InHAL] -> Bus Selector36/1
  [VINP_Obstacle8_BUS_InHAL] -> Bus Selector37/1
  [VINP_Obstacle9_BUS_InHAL] -> Bus Selector38/1
  [VINP_Obstacle10_BUS_InHAL] -> Bus Selector39/1
  Bus Selector39/1 -> Mux3/10  "<VINP_CamObs10PosXValid_flg_InHAL>"
  Mux3/1 -> VINP_CamObsPosXValid10_flg_InProc/1  "VINP_CamObsPosXValid10_flg_InProc"
  Bus Selector40/1 -> Mux4/1  "<VINP_CamObs1PosY_m_InHAL>"
  Bus Selector41/1 -> Mux4/2  "<VINP_CamObs2PosY_m_InHAL>"
  Bus Selector42/1 -> Mux4/3  "<VINP_CamObs3PosY_m_InHAL>"
  Bus Selector43/1 -> Mux4/4  "<VINP_CamObs4PosY_m_InHAL>"
  Bus Selector44/1 -> Mux4/5  "<VINP_CamObs5PosY_m_InHAL>"
  Bus Selector45/1 -> Mux4/6  "<VINP_CamObs6PosY_m_InHAL>"
  Bus Selector46/1 -> Mux4/7  "<VINP_CamObs7PosY_m_InHAL>"
  Bus Selector47/1 -> Mux4/8  "<VINP_CamObs8PosY_m_InHAL>"
  Bus Selector48/1 -> Mux4/9  "<VINP_CamObs9PosY_m_InHAL>"
  [VINP_Obstacle1_BUS_InHAL] -> Bus Selector40/1
  [VINP_Obstacle2_BUS_InHAL] -> Bus Selector41/1
  [VINP_Obstacle3_BUS_InHAL] -> Bus Selector42/1
  [VINP_Obstacle4_BUS_InHAL] -> Bus Selector43/1
  [VINP_Obstacle5_BUS_InHAL] -> Bus Selector44/1
  [VINP_Obstacle6_BUS_InHAL] -> Bus Selector45/1
  [VINP_Obstacle7_BUS_InHAL] -> Bus Selector46/1
  [VINP_Obstacle8_BUS_InHAL] -> Bus Selector47/1
  [VINP_Obstacle9_BUS_InHAL] -> Bus Selector48/1
  [VINP_Obstacle10_BUS_InHAL] -> Bus Selector49/1
  Bus Selector49/1 -> Mux4/10  "<VINP_CamObs10PosY_m_InHAL>"
  Mux4/1 -> VINP_CamObsPosY10_m_InProc/1  "VINP_CamObsPosY10_m_InProc"
  Bus Selector50/1 -> Mux5/1  "<VINP_CamObs1PosYValid_flg_InHAL>"
  Bus Selector51/1 -> Mux5/2  "<VINP_CamObs2PosYValid_flg_InHAL>"
  Bus Selector52/1 -> Mux5/3  "<VINP_CamObs3PosYValid_flg_InHAL>"
  Bus Selector53/1 -> Mux5/4  "<VINP_CamObs4PosYValid_flg_InHAL>"
  Bus Selector54/1 -> Mux5/5  "<VINP_CamObs5PosYValid_flg_InHAL>"
  Bus Selector55/1 -> Mux5/6  "<VINP_CamObs6PosYValid_flg_InHAL>"
  Bus Selector56/1 -> Mux5/7  "<VINP_CamObs7PosYValid_flg_InHAL>"
  Bus Selector57/1 -> Mux5/8  "<VINP_CamObs8PosYValid_flg_InHAL>"
  Bus Selector58/1 -> Mux5/9  "<VINP_CamObs9PosYValid_flg_InHAL>"
  [VINP_Obstacle1_BUS_InHAL] -> Bus Selector50/1
  [VINP_Obstacle2_BUS_InHAL] -> Bus Selector51/1
  [VINP_Obstacle3_BUS_InHAL] -> Bus Selector52/1
  [VINP_Obstacle4_BUS_InHAL] -> Bus Selector53/1
  [VINP_Obstacle5_BUS_InHAL] -> Bus Selector54/1